simulink library "inp"  (Simulink 8.2)
settings: Solver=ode45  SolverMode=SingleTasking  StartTime=0.0  StopTime=10.0  RelTol=1e-3
undefined symbols (not defined in the file or in its folder): C_TICK_TIME_S, ENUM_FAULTSTATE_FAIL, ENUM_FAULTSTATE_INDETERMINATE, ENUM_FAULTSTATE_PASS, ENUM_KEYSWITCH_ON, FALSE, KINP_ABS1CANCommuOk_flg_ovrdflg, KINP_ABS1CANCommuOk_flg_ovrdval, KINP_ABS1CANPeriodTime_s, KINP_ABS1CANRolCntOk_flg_ovrdflg, KINP_ABS1CANRolCntOk_flg_ovrdval, KINP_ABS2CANChkSumOk_flg_ovrdflg, KINP_ABS2CANChkSumOk_flg_ovrdval, KINP_ABS2CANCommuOk_flg_ovrdflg, KINP_ABS2CANCommuOk_flg_ovrdval, KINP_ABS2CANPeriodTime_s, KINP_ABS2CANRolCntOk_flg_ovrdflg, KINP_ABS2CANRolCntOk_flg_ovrdval, KINP_ABS3CANCommuOk_flg_ovrdflg, KINP_ABS3CANCommuOk_flg_ovrdval, KINP_ABS4CANCommuOk_flg_ovrdflg, KINP_ABS4CANCommuOk_flg_ovrdval, KINP_ABSActive_flg_defval, KINP_ABSActive_flg_invalid, KINP_ABSActive_flg_ovrdflg, KINP_ABSActive_flg_ovrdval, KINP_ABSActive_flg_setvalid, KINP_ABSActive_flg_srvcflg, KINP_ABSActive_flg_srvcval, KINP_ABSCANAlive_flg_ovrdflg, KINP_ABSCANAlive_flg_ovrdval, KINP_ABSCANCommuOk_flg_ovrdflg, KINP_ABSCANCommuOk_flg_ovrdval, KINP_ABSCANValidOk_flg_ovrdflg, KINP_ABSCANValidOk_flg_ovrdval, KINP_ABSFault_flg_defval, KINP_ABSFault_flg_invalid, KINP_ABSFault_flg_ovrdflg, KINP_ABSFault_flg_ovrdval, KINP_ABSFault_flg_setvalid, KINP_ABSFault_flg_srvcflg, KINP_ABSFault_flg_srvcval, KINP_AC1CANCommuOk_flg_ovrdflg, KINP_AC1CANCommuOk_flg_ovrdval, KINP_ACCANAlive_flg_ovrdflg, KINP_ACCANAlive_flg_ovrdval, KINP_ACCANCommuOk_flg_ovrdflg, KINP_ACCANCommuOk_flg_ovrdval, KINP_ACCANValidOk_flg_ovrdflg, KINP_ACCANValidOk_flg_ovrdval, KINP_ACCCancelH_flg_defval, KINP_ACCCancelH_flg_invalid, KINP_ACCCancelH_flg_ovrdflg, KINP_ACCCancelH_flg_ovrdval, KINP_ACCCancelH_flg_setvalid, KINP_ACCCancelH_flg_srvcflg, KINP_ACCCancelH_flg_srvcval, KINP_AccelPedalPosn_pct_defval, KINP_AccelPedalPosn_pct_gain, KINP_AccelPedalPosn_pct_invalid ... (+1866)
libraries: inp x192, hcu_lib x34, simulink x33
(part 12 of 21)

== system inp/INP/EPM_signals/TimeToLaneCrossingRight ==
  signal_in : Inport
  msg_valid : Inport  Port=2
  Active_fault_flg : Inport  Port=3
  can_signal_process_wio_validity : inp/can_signal_process_wio_validity
  conv_gain_offset : Subsystem
  defval : Constant  Value=KINP_TLCR_s_defval
  invalid : Constant  Value=KINP_TLCR_s_invalid
  min_max_limit : Subsystem
  ovrdflg : Constant  Value=KINP_TLCR_s_ovrdflg
  ovrdval : Constant  Value=KINP_TLCR_s_ovrdval
  setvalid : Constant  Value=KINP_TLCR_s_setvalid
  srvcflg : Constant  Value=KINP_TLCR_s_srvcflg
  srvcval : Constant  Value=KINP_TLCR_s_srvcval
  signal_out : Outport
  signal_valid_out : Outport  Port=2
  wires:
  Active_fault_flg/1 -> can_signal_process_wio_validity/3
  srvcval/1 -> can_signal_process_wio_validity/10
  srvcflg/1 -> can_signal_process_wio_validity/9
  ovrdval/1 -> can_signal_process_wio_validity/8
  ovrdflg/1 -> can_signal_process_wio_validity/7
  setvalid/1 -> can_signal_process_wio_validity/6
  min_max_limit/1(out) -> signal_out/1
  conv_gain_offset/1(out) -> can_signal_process_wio_validity/1
  invalid/1 -> can_signal_process_wio_validity/5
  defval/1 -> can_signal_process_wio_validity/4
  msg_valid/1 -> can_signal_process_wio_validity/2
  signal_in/1 -> conv_gain_offset/1(in)
  can_signal_process_wio_validity/2 -> signal_valid_out/1
  can_signal_process_wio_validity/1 -> min_max_limit/1(in)

== system inp/INP/EPM_signals/ViewRangeStartL ==
  signal_in : Inport
  msg_valid : Inport  Port=2
  Active_fault_flg : Inport  Port=3
  can_signal_process_wio_validity : inp/can_signal_process_wio_validity
  conv_gain_offset : Subsystem
  defval : Constant  Value=KINP_ViewRangeStartL_m_defval
  invalid : Constant  Value=KINP_ViewRangeStartL_m_invalid
  min_max_limit : Subsystem
  ovrdflg : Constant  Value=KINP_ViewRangeStartL_m_ovrdflg
  ovrdval : Constant  Value=KINP_ViewRangeStartL_m_ovrdval
  setvalid : Constant  Value=KINP_ViewRangeStartL_m_setvalid
  srvcflg : Constant  Value=KINP_ViewRangeStartL_m_srvcflg
  srvcval : Constant  Value=KINP_ViewRangeStartL_m_srvcval
  signal_out : Outport
  signal_valid_out : Outport  Port=2
  wires:
  Active_fault_flg/1 -> can_signal_process_wio_validity/3
  srvcval/1 -> can_signal_process_wio_validity/10
  srvcflg/1 -> can_signal_process_wio_validity/9
  ovrdval/1 -> can_signal_process_wio_validity/8
  ovrdflg/1 -> can_signal_process_wio_validity/7
  setvalid/1 -> can_signal_process_wio_validity/6
  min_max_limit/1(out) -> signal_out/1
  conv_gain_offset/1(out) -> can_signal_process_wio_validity/1
  invalid/1 -> can_signal_process_wio_validity/5
  defval/1 -> can_signal_process_wio_validity/4
  msg_valid/1 -> can_signal_process_wio_validity/2
  signal_in/1 -> conv_gain_offset/1(in)
  can_signal_process_wio_validity/2 -> signal_valid_out/1
  can_signal_process_wio_validity/1 -> min_max_limit/1(in)

== system inp/INP/EPM_signals/ViewRangeStartR ==
  signal_in : Inport
  msg_valid : Inport  Port=2
  Active_fault_flg : Inport  Port=3
  can_signal_process_wio_validity : inp/can_signal_process_wio_validity
  conv_gain_offset : Subsystem
  defval : Constant  Value=KINP_ViewRangeStartR_m_defval
  invalid : Constant  Value=KINP_ViewRangeStartR_m_invalid
  min_max_limit : Subsystem
  ovrdflg : Constant  Value=KINP_ViewRangeStartR_m_ovrdflg
  ovrdval : Constant  Value=KINP_ViewRangeStartR_m_ovrdval
  setvalid : Constant  Value=KINP_ViewRangeStartR_m_setvalid
  srvcflg : Constant  Value=KINP_ViewRangeStartR_m_srvcflg
  srvcval : Constant  Value=KINP_ViewRangeStartR_m_srvcval
  signal_out : Outport
  signal_valid_out : Outport  Port=2
  wires:
  can_signal_process_wio_validity/1 -> min_max_limit/1(in)
  can_signal_process_wio_validity/2 -> signal_valid_out/1
  signal_in/1 -> conv_gain_offset/1(in)
  msg_valid/1 -> can_signal_process_wio_validity/2
  defval/1 -> can_signal_process_wio_validity/4
  invalid/1 -> can_signal_process_wio_validity/5
  conv_gain_offset/1(out) -> can_signal_process_wio_validity/1
  min_max_limit/1(out) -> signal_out/1
  setvalid/1 -> can_signal_process_wio_validity/6
  ovrdflg/1 -> can_signal_process_wio_validity/7
  ovrdval/1 -> can_signal_process_wio_validity/8
  srvcflg/1 -> can_signal_process_wio_validity/9
  srvcval/1 -> can_signal_process_wio_validity/10
  Active_fault_flg/1 -> can_signal_process_wio_validity/3

== system inp/INP/EPS_signals/AckCurStrAngReq ==
  signal_in : Inport
  signal_valid_in : Inport  Port=2
  msg_valid : Inport  Port=3
  Active_fault_flg : Inport  Port=4
  can_signal_process_with_validity : inp/can_signal_process_with_validity
  conv_gain_offset : Subsystem
  defval : Constant  Value=KINP_AckCurStrAngReq_deg_defval
  invalid : Constant  Value=KINP_AckCurStrAngReq_deg_invalid
  min_max_limit : Subsystem
  ovrdflg : Constant  Value=KINP_AckCurStrAngReq_deg_ovrdflg
  ovrdval : Constant  Value=KINP_AckCurStrAngReq_deg_ovrdval
  setvalid : Constant  Value=KINP_AckCurStrAngReq_deg_setvalid
  srvcflg : Constant  Value=KINP_AckCurStrAngReq_deg_srvcflg
  srvcval : Constant  Value=KINP_AckCurStrAngReq_deg_srvcval
  signal_out : Outport
  signal_valid_out : Outport  Port=2
  raw_signal_valid_out : Outport  Port=3
  wires:
  can_signal_process_with_validity/3 -> raw_signal_valid_out/1
  Active_fault_flg/1 -> can_signal_process_with_validity/4
  srvcval/1 -> can_signal_process_with_validity/12
  srvcflg/1 -> can_signal_process_with_validity/11
  ovrdval/1 -> can_signal_process_with_validity/10
  ovrdflg/1 -> can_signal_process_with_validity/9
  setvalid/1 -> can_signal_process_with_validity/8
  min_max_limit/1(out) -> signal_out/1
  conv_gain_offset/1(out) -> can_signal_process_with_validity/1
  invalid/1 -> can_signal_process_with_validity/7
  defval/1 -> can_signal_process_with_validity/6
  msg_valid/1 -> can_signal_process_with_validity/5, can_signal_process_with_validity/3
  signal_valid_in/1 -> can_signal_process_with_validity/2
  signal_in/1 -> conv_gain_offset/1(in)
  can_signal_process_with_validity/2 -> signal_valid_out/1
  can_signal_process_with_validity/1 -> min_max_limit/1(in)

== system inp/INP/EPS_signals/AckCurStrAngReqApplable ==
  signal_in : Inport
  msg_valid : Inport  Port=2
  Active_fault_flg : Inport  Port=3
  can_signal_process_wio_validity : inp/can_signal_process_wio_validity
  defval : Constant  Value=KINP_AckCurStrAngReqApplable_flg_defval
  invalid : Constant  Value=KINP_AckCurStrAngReqApplable_flg_invalid
  ovrdflg : Constant  Value=KINP_AckCurStrAngReqApplable_flg_ovrdflg
  ovrdval : Constant  Value=KINP_AckCurStrAngReqApplable_flg_ovrdval
  setvalid : Constant  Value=KINP_AckCurStrAngReqApplable_flg_setvalid
  srvcflg : Constant  Value=KINP_AckCurStrAngReqApplable_flg_srvcflg
  srvcval : Constant  Value=KINP_AckCurStrAngReqApplable_flg_srvcval
  signal_out : Outport
  signal_valid_out : Outport  Port=2
  wires:
  Active_fault_flg/1 -> can_signal_process_wio_validity/3
  srvcval/1 -> can_signal_process_wio_validity/10
  srvcflg/1 -> can_signal_process_wio_validity/9
  ovrdval/1 -> can_signal_process_wio_validity/8
  ovrdflg/1 -> can_signal_process_wio_validity/7
  setvalid/1 -> can_signal_process_wio_validity/6
  invalid/1 -> can_signal_process_wio_validity/5
  defval/1 -> can_signal_process_wio_validity/4
  msg_valid/1 -> can_signal_process_wio_validity/2
  signal_in/1 -> can_signal_process_wio_validity/1
  can_signal_process_wio_validity/2 -> signal_valid_out/1
  can_signal_process_wio_validity/1 -> signal_out/1

== system inp/INP/EPS_signals/AckCurStrTqReq ==
  signal_in : Inport
  signal_valid_in : Inport  Port=2
  msg_valid : Inport  Port=3
  Active_fault_flg : Inport  Port=4
  can_signal_process_with_validity : inp/can_signal_process_with_validity
  conv_gain_offset : Subsystem
  defval : Constant  Value=KINP_AckCurStrTqReq_Nm_defval
  invalid : Constant  Value=KINP_AckCurStrTqReq_Nm_invalid
  min_max_limit : Subsystem
  ovrdflg : Constant  Value=KINP_AckCurStrTqReq_Nm_ovrdflg
  ovrdval : Constant  Value=KINP_AckCurStrTqReq_Nm_ovrdval
  setvalid : Constant  Value=KINP_AckCurStrTqReq_Nm_setvalid
  srvcflg : Constant  Value=KINP_AckCurStrTqReq_Nm_srvcflg
  srvcval : Constant  Value=KINP_AckCurStrTqReq_Nm_srvcval
  signal_out : Outport
  signal_valid_out : Outport  Port=2
  raw_signal_valid_out : Outport  Port=3
  wires:
  can_signal_process_with_validity/1 -> min_max_limit/1(in)
  can_signal_process_with_validity/2 -> signal_valid_out/1
  signal_in/1 -> conv_gain_offset/1(in)
  signal_valid_in/1 -> can_signal_process_with_validity/2
  msg_valid/1 -> can_signal_process_with_validity/3, can_signal_process_with_validity/5
  defval/1 -> can_signal_process_with_validity/6
  invalid/1 -> can_signal_process_with_validity/7
  conv_gain_offset/1(out) -> can_signal_process_with_validity/1
  min_max_limit/1(out) -> signal_out/1
  setvalid/1 -> can_signal_process_with_validity/8
  ovrdflg/1 -> can_signal_process_with_validity/9
  ovrdval/1 -> can_signal_process_with_validity/10
  srvcflg/1 -> can_signal_process_with_validity/11
  srvcval/1 -> can_signal_process_with_validity/12
  Active_fault_flg/1 -> can_signal_process_with_validity/4
  can_signal_process_with_validity/3 -> raw_signal_valid_out/1

== system inp/INP/EPS_signals/AckCurStrTqReqApplable ==
  signal_in : Inport
  msg_valid : Inport  Port=2
  Active_fault_flg : Inport  Port=3
  can_signal_process_wio_validity : inp/can_signal_process_wio_validity
  defval : Constant  Value=KINP_AckCurStrTqReqApplable_flg_defval
  invalid : Constant  Value=KINP_AckCurStrTqReqApplable_flg_invalid
  ovrdflg : Constant  Value=KINP_AckCurStrTqReqApplable_flg_ovrdflg
  ovrdval : Constant  Value=KINP_AckCurStrTqReqApplable_flg_ovrdval
  setvalid : Constant  Value=KINP_AckCurStrTqReqApplable_flg_setvalid
  srvcflg : Constant  Value=KINP_AckCurStrTqReqApplable_flg_srvcflg
  srvcval : Constant  Value=KINP_AckCurStrTqReqApplable_flg_srvcval
  signal_out : Outport
  signal_valid_out : Outport  Port=2
  wires:
  can_signal_process_wio_validity/1 -> signal_out/1
  can_signal_process_wio_validity/2 -> signal_valid_out/1
  signal_in/1 -> can_signal_process_wio_validity/1
  msg_valid/1 -> can_signal_process_wio_validity/2
  defval/1 -> can_signal_process_wio_validity/4
  invalid/1 -> can_signal_process_wio_validity/5
  setvalid/1 -> can_signal_process_wio_validity/6
  ovrdflg/1 -> can_signal_process_wio_validity/7
  ovrdval/1 -> can_signal_process_wio_validity/8
  srvcflg/1 -> can_signal_process_wio_validity/9
  srvcval/1 -> can_signal_process_wio_validity/10
  Active_fault_flg/1 -> can_signal_process_wio_validity/3

== system inp/INP/EPS_signals/EPASMILReq ==
  signal_in : Inport
  msg_valid : Inport  Port=2
  Active_fault_flg : Inport  Port=3
  can_signal_process_wio_validity : inp/can_signal_process_wio_validity
  defval : Constant  Value=KINP_EPASMILReq_flg_defval
  invalid : Constant  Value=KINP_EPASMILReq_flg_invalid
  ovrdflg : Constant  Value=KINP_EPASMILReq_flg_ovrdflg
  ovrdval : Constant  Value=KINP_EPASMILReq_flg_ovrdval
  setvalid : Constant  Value=KINP_EPASMILReq_flg_setvalid
  srvcflg : Constant  Value=KINP_EPASMILReq_flg_srvcflg
  srvcval : Constant  Value=KINP_EPASMILReq_flg_srvcval
  signal_out : Outport
  signal_valid_out : Outport  Port=2
  wires:
  Active_fault_flg/1 -> can_signal_process_wio_validity/3
  srvcval/1 -> can_signal_process_wio_validity/10
  srvcflg/1 -> can_signal_process_wio_validity/9
  ovrdval/1 -> can_signal_process_wio_validity/8
  ovrdflg/1 -> can_signal_process_wio_validity/7
  setvalid/1 -> can_signal_process_wio_validity/6
  invalid/1 -> can_signal_process_wio_validity/5
  defval/1 -> can_signal_process_wio_validity/4
  msg_valid/1 -> can_signal_process_wio_validity/2
  signal_in/1 -> can_signal_process_wio_validity/1
  can_signal_process_wio_validity/2 -> signal_valid_out/1
  can_signal_process_wio_validity/1 -> signal_out/1

== system inp/INP/EPS_signals/EPSBattVolt ==
  signal_in : Inport
  signal_valid_in : Inport  Port=2
  msg_valid : Inport  Port=3
  Active_fault_flg : Inport  Port=4
  can_signal_process_with_validity : inp/can_signal_process_with_validity
  conv_gain_offset : Subsystem
  defval : Constant  Value=KINP_EPSBattVolt_V_defval
  invalid : Constant  Value=KINP_EPSBattVolt_V_invalid
  min_max_limit : Subsystem
  ovrdflg : Constant  Value=KINP_EPSBattVolt_V_ovrdflg
  ovrdval : Constant  Value=KINP_EPSBattVolt_V_ovrdval
  setvalid : Constant  Value=KINP_EPSBattVolt_V_setvalid
  srvcflg : Constant  Value=KINP_EPSBattVolt_V_srvcflg
  srvcval : Constant  Value=KINP_EPSBattVolt_V_srvcval
  signal_out : Outport
  signal_valid_out : Outport  Port=2
  raw_signal_valid_out : Outport  Port=3
  wires:
  can_signal_process_with_validity/3 -> raw_signal_valid_out/1
  Active_fault_flg/1 -> can_signal_process_with_validity/4
  srvcval/1 -> can_signal_process_with_validity/12
  srvcflg/1 -> can_signal_process_with_validity/11
  ovrdval/1 -> can_signal_process_with_validity/10
  ovrdflg/1 -> can_signal_process_with_validity/9
  setvalid/1 -> can_signal_process_with_validity/8
  min_max_limit/1(out) -> signal_out/1
  conv_gain_offset/1(out) -> can_signal_process_with_validity/1
  invalid/1 -> can_signal_process_with_validity/7
  defval/1 -> can_signal_process_with_validity/6
  msg_valid/1 -> can_signal_process_with_validity/5, can_signal_process_with_validity/3
  signal_valid_in/1 -> can_signal_process_with_validity/2
  signal_in/1 -> conv_gain_offset/1(in)
  can_signal_process_with_validity/2 -> signal_valid_out/1
  can_signal_process_with_validity/1 -> min_max_limit/1(in)

== system inp/INP/EPS_signals/EPSElectPWR ==
  signal_in : Inport
  msg_valid : Inport  Port=2
  Active_fault_flg : Inport  Port=3
  can_signal_process_wio_validity : inp/can_signal_process_wio_validity
  conv_gain_offset : Subsystem
  defval : Constant  Value=KINP_EPSElectPWR_A_defval
  invalid : Constant  Value=KINP_EPSElectPWR_A_invalid
  min_max_limit : Subsystem
  ovrdflg : Constant  Value=KINP_EPSElectPWR_A_ovrdflg
  ovrdval : Constant  Value=KINP_EPSElectPWR_A_ovrdval
  setvalid : Constant  Value=KINP_EPSElectPWR_A_setvalid
  srvcflg : Constant  Value=KINP_EPSElectPWR_A_srvcflg
  srvcval : Constant  Value=KINP_EPSElectPWR_A_srvcval
  signal_out : Outport
  signal_valid_out : Outport  Port=2
  wires:
  can_signal_process_wio_validity/1 -> min_max_limit/1(in)
  can_signal_process_wio_validity/2 -> signal_valid_out/1
  signal_in/1 -> conv_gain_offset/1(in)
  msg_valid/1 -> can_signal_process_wio_validity/2
  defval/1 -> can_signal_process_wio_validity/4
  invalid/1 -> can_signal_process_wio_validity/5
  conv_gain_offset/1(out) -> can_signal_process_wio_validity/1
  min_max_limit/1(out) -> signal_out/1
  setvalid/1 -> can_signal_process_wio_validity/6
  ovrdflg/1 -> can_signal_process_wio_validity/7
  ovrdval/1 -> can_signal_process_wio_validity/8
  srvcflg/1 -> can_signal_process_wio_validity/9
  srvcval/1 -> can_signal_process_wio_validity/10
  Active_fault_flg/1 -> can_signal_process_wio_validity/3

== system inp/INP/EPS_signals/EPSFailed ==
  signal_in : Inport
  msg_valid : Inport  Port=2
  Active_fault_flg : Inport  Port=3
  can_signal_process_wio_validity : inp/can_signal_process_wio_validity
  defval : Constant  Value=KINP_EPSFailed_flg_defval
  invalid : Constant  Value=KINP_EPSFailed_flg_invalid
  ovrdflg : Constant  Value=KINP_EPSFailed_flg_ovrdflg
  ovrdval : Constant  Value=KINP_EPSFailed_flg_ovrdval
  setvalid : Constant  Value=KINP_EPSFailed_flg_setvalid
  srvcflg : Constant  Value=KINP_EPSFailed_flg_srvcflg
  srvcval : Constant  Value=KINP_EPSFailed_flg_srvcval
  signal_out : Outport
  signal_valid_out : Outport  Port=2
  wires:
  Active_fault_flg/1 -> can_signal_process_wio_validity/3
  srvcval/1 -> can_signal_process_wio_validity/10
  srvcflg/1 -> can_signal_process_wio_validity/9
  ovrdval/1 -> can_signal_process_wio_validity/8
  ovrdflg/1 -> can_signal_process_wio_validity/7
  setvalid/1 -> can_signal_process_wio_validity/6
  invalid/1 -> can_signal_process_wio_validity/5
  defval/1 -> can_signal_process_wio_validity/4
  msg_valid/1 -> can_signal_process_wio_validity/2
  signal_in/1 -> can_signal_process_wio_validity/1
  can_signal_process_wio_validity/2 -> signal_valid_out/1
  can_signal_process_wio_validity/1 -> signal_out/1

== system inp/INP/EPS_signals/RelSteerAngle ==
  signal_in : Inport
  signal_valid_in : Inport  Port=2
  msg_valid : Inport  Port=3
  Active_fault_flg : Inport  Port=4
  can_signal_process_with_validity : inp/can_signal_process_with_validity
  conv_gain_offset : Subsystem
  defval : Constant  Value=KINP_RelSteerAngle_deg_defval
  invalid : Constant  Value=KINP_RelSteerAngle_deg_invalid
  min_max_limit : Subsystem
  ovrdflg : Constant  Value=KINP_RelSteerAngle_deg_ovrdflg
  ovrdval : Constant  Value=KINP_RelSteerAngle_deg_ovrdval
  setvalid : Constant  Value=KINP_RelSteerAngle_deg_setvalid
  srvcflg : Constant  Value=KINP_RelSteerAngle_deg_srvcflg
  srvcval : Constant  Value=KINP_RelSteerAngle_deg_srvcval
  signal_out : Outport
  signal_valid_out : Outport  Port=2
  raw_signal_valid_out : Outport  Port=3
  wires:
  can_signal_process_with_validity/1 -> min_max_limit/1(in)
  can_signal_process_with_validity/2 -> signal_valid_out/1
  signal_in/1 -> conv_gain_offset/1(in)
  signal_valid_in/1 -> can_signal_process_with_validity/2
  msg_valid/1 -> can_signal_process_with_validity/3, can_signal_process_with_validity/5
  defval/1 -> can_signal_process_with_validity/6
  invalid/1 -> can_signal_process_with_validity/7
  conv_gain_offset/1(out) -> can_signal_process_with_validity/1
  min_max_limit/1(out) -> signal_out/1
  setvalid/1 -> can_signal_process_with_validity/8
  ovrdflg/1 -> can_signal_process_with_validity/9
  ovrdval/1 -> can_signal_process_with_validity/10
  srvcflg/1 -> can_signal_process_with_validity/11
  srvcval/1 -> can_signal_process_with_validity/12
  Active_fault_flg/1 -> can_signal_process_with_validity/4
  can_signal_process_with_validity/3 -> raw_signal_valid_out/1

== system inp/INP/EPS_signals/SteerTorque ==
  signal_in : Inport
  signal_valid_in : Inport  Port=2
  msg_valid : Inport  Port=3
  Active_fault_flg : Inport  Port=4
  can_signal_process_with_validity : inp/can_signal_process_with_validity
  conv_gain_offset : Subsystem
  defval : Constant  Value=KINP_SteerTorque_Nm_defval
  invalid : Constant  Value=KINP_SteerTorque_Nm_invalid
  min_max_limit : Subsystem
  ovrdflg : Constant  Value=KINP_SteerTorque_Nm_ovrdflg
  ovrdval : Constant  Value=KINP_SteerTorque_Nm_ovrdval
  setvalid : Constant  Value=KINP_SteerTorque_Nm_setvalid
  srvcflg : Constant  Value=KINP_SteerTorque_Nm_srvcflg
  srvcval : Constant  Value=KINP_SteerTorque_Nm_srvcval
  signal_out : Outport
  signal_valid_out : Outport  Port=2
  raw_signal_valid_out : Outport  Port=3
  wires:
  can_signal_process_with_validity/3 -> raw_signal_valid_out/1
  Active_fault_flg/1 -> can_signal_process_with_validity/4
  srvcval/1 -> can_signal_process_with_validity/12
  srvcflg/1 -> can_signal_process_with_validity/11
  ovrdval/1 -> can_signal_process_with_validity/10
  ovrdflg/1 -> can_signal_process_with_validity/9
  setvalid/1 -> can_signal_process_with_validity/8
  min_max_limit/1(out) -> signal_out/1
  conv_gain_offset/1(out) -> can_signal_process_with_validity/1
  invalid/1 -> can_signal_process_with_validity/7
  defval/1 -> can_signal_process_with_validity/6
  msg_valid/1 -> can_signal_process_with_validity/5, can_signal_process_with_validity/3
  signal_valid_in/1 -> can_signal_process_with_validity/2
  signal_in/1 -> conv_gain_offset/1(in)
  can_signal_process_with_validity/2 -> signal_valid_out/1
  can_signal_process_with_validity/1 -> min_max_limit/1(in)

== system inp/INP/EPS_signals/SteeringAngle ==
  signal_in : Inport
  signal_valid_in : Inport  Port=2
  msg_valid : Inport  Port=3
  Active_fault_flg : Inport  Port=4
  can_signal_process_with_validity : inp/can_signal_process_with_validity
  conv_gain_offset : Subsystem
  defval : Constant  Value=KINP_SteerAngle_deg_defval
  invalid : Constant  Value=KINP_SteerAngle_deg_invalid
  min_max_limit : Subsystem
  ovrdflg : Constant  Value=KINP_SteerAngle_deg_ovrdflg
  ovrdval : Constant  Value=KINP_SteerAngle_deg_ovrdval
  setvalid : Constant  Value=KINP_SteerAngle_deg_setvalid
  srvcflg : Constant  Value=KINP_SteerAngle_deg_srvcflg
  srvcval : Constant  Value=KINP_SteerAngle_deg_srvcval
  signal_out : Outport
  signal_valid_out : Outport  Port=2
  raw_signal_valid_out : Outport  Port=3
  wires:
  can_signal_process_with_validity/1 -> min_max_limit/1(in)
  can_signal_process_with_validity/2 -> signal_valid_out/1
  signal_in/1 -> conv_gain_offset/1(in)
  signal_valid_in/1 -> can_signal_process_with_validity/2
  msg_valid/1 -> can_signal_process_with_validity/3, can_signal_process_with_validity/5
  defval/1 -> can_signal_process_with_validity/6
  invalid/1 -> can_signal_process_with_validity/7
  conv_gain_offset/1(out) -> can_signal_process_with_validity/1
  min_max_limit/1(out) -> signal_out/1
  setvalid/1 -> can_signal_process_with_validity/8
  ovrdflg/1 -> can_signal_process_with_validity/9
  ovrdval/1 -> can_signal_process_with_validity/10
  srvcflg/1 -> can_signal_process_with_validity/11
  srvcval/1 -> can_signal_process_with_validity/12
  Active_fault_flg/1 -> can_signal_process_with_validity/4
  can_signal_process_with_validity/3 -> raw_signal_valid_out/1

== system inp/INP/EPS_signals/SteeringSpeed ==
  signal_in : Inport
  signal_valid_in : Inport  Port=2
  msg_valid : Inport  Port=3
  Active_fault_flg : Inport  Port=4
  can_signal_process_with_validity : inp/can_signal_process_with_validity
  conv_gain_offset : Subsystem
  defval : Constant  Value=KINP_SteerSpeed_degps_defval
  invalid : Constant  Value=KINP_SteerSpeed_degps_invalid
  min_max_limit : Subsystem
  ovrdflg : Constant  Value=KINP_SteerSpeed_degps_ovrdflg
  ovrdval : Constant  Value=KINP_SteerSpeed_degps_ovrdval
  setvalid : Constant  Value=KINP_SteerSpeed_degps_setvalid
  srvcflg : Constant  Value=KINP_SteerSpeed_degps_srvcflg
  srvcval : Constant  Value=KINP_SteerSpeed_degps_srvcval
  signal_out : Outport
  signal_valid_out : Outport  Port=2
  raw_signal_valid_out : Outport  Port=3
  wires:
  can_signal_process_with_validity/3 -> raw_signal_valid_out/1
  Active_fault_flg/1 -> can_signal_process_with_validity/4
  srvcval/1 -> can_signal_process_with_validity/12
  srvcflg/1 -> can_signal_process_with_validity/11
  ovrdval/1 -> can_signal_process_with_validity/10
  ovrdflg/1 -> can_signal_process_with_validity/9
  setvalid/1 -> can_signal_process_with_validity/8
  min_max_limit/1(out) -> signal_out/1
  conv_gain_offset/1(out) -> can_signal_process_with_validity/1
  invalid/1 -> can_signal_process_with_validity/7
  defval/1 -> can_signal_process_with_validity/6
  msg_valid/1 -> can_signal_process_with_validity/5, can_signal_process_with_validity/3
  signal_valid_in/1 -> can_signal_process_with_validity/2
  signal_in/1 -> conv_gain_offset/1(in)
  can_signal_process_with_validity/2 -> signal_valid_out/1
  can_signal_process_with_validity/1 -> min_max_limit/1(in)

== system inp/INP/HMI_signals/ACCCancelH ==
  signal_in : Inport
  msg_valid : Inport  Port=2
  Active_fault_flg : Inport  Port=3
  can_signal_process_wio_validity : inp/can_signal_process_wio_validity
  defval : Constant  Value=KINP_ACCCancelH_flg_defval
  invalid : Constant  Value=KINP_ACCCancelH_flg_invalid
  ovrdflg : Constant  Value=KINP_ACCCancelH_flg_ovrdflg
  ovrdval : Constant  Value=KINP_ACCCancelH_flg_ovrdval
  setvalid : Constant  Value=KINP_ACCCancelH_flg_setvalid
  srvcflg : Constant  Value=KINP_ACCCancelH_flg_srvcflg
  srvcval : Constant  Value=KINP_ACCCancelH_flg_srvcval
  signal_out : Outport
  signal_valid_out : Outport  Port=2
  wires:
  Active_fault_flg/1 -> can_signal_process_wio_validity/3
  srvcval/1 -> can_signal_process_wio_validity/10
  srvcflg/1 -> can_signal_process_wio_validity/9
  ovrdval/1 -> can_signal_process_wio_validity/8
  ovrdflg/1 -> can_signal_process_wio_validity/7
  setvalid/1 -> can_signal_process_wio_validity/6
  invalid/1 -> can_signal_process_wio_validity/5
  defval/1 -> can_signal_process_wio_validity/4
  msg_valid/1 -> can_signal_process_wio_validity/2
  signal_in/1 -> can_signal_process_wio_validity/1
  can_signal_process_wio_validity/2 -> signal_valid_out/1
  can_signal_process_wio_validity/1 -> signal_out/1

== system inp/INP/HMI_signals/ACCOnH ==
  signal_in : Inport
  msg_valid : Inport  Port=2
  Active_fault_flg : Inport  Port=3
  can_signal_process_wio_validity : inp/can_signal_process_wio_validity
  defval : Constant  Value=KINP_ACCOnH_flg_defval
  invalid : Constant  Value=KINP_ACCOnH_flg_invalid
  ovrdflg : Constant  Value=KINP_ACCOnH_flg_ovrdflg
  ovrdval : Constant  Value=KINP_ACCOnH_flg_ovrdval
  setvalid : Constant  Value=KINP_ACCOnH_flg_setvalid
  srvcflg : Constant  Value=KINP_ACCOnH_flg_srvcflg
  srvcval : Constant  Value=KINP_ACCOnH_flg_srvcval
  signal_out : Outport
  signal_valid_out : Outport  Port=2
  wires:
  can_signal_process_wio_validity/1 -> signal_out/1
  can_signal_process_wio_validity/2 -> signal_valid_out/1
  signal_in/1 -> can_signal_process_wio_validity/1
  msg_valid/1 -> can_signal_process_wio_validity/2
  defval/1 -> can_signal_process_wio_validity/4
  invalid/1 -> can_signal_process_wio_validity/5
  setvalid/1 -> can_signal_process_wio_validity/6
  ovrdflg/1 -> can_signal_process_wio_validity/7
  ovrdval/1 -> can_signal_process_wio_validity/8
  srvcflg/1 -> can_signal_process_wio_validity/9
  srvcval/1 -> can_signal_process_wio_validity/10
  Active_fault_flg/1 -> can_signal_process_wio_validity/3

== system inp/INP/HMI_signals/ACCResSpdIncH ==
  signal_in : Inport
  msg_valid : Inport  Port=2
  Active_fault_flg : Inport  Port=3
  can_signal_process_wio_validity : inp/can_signal_process_wio_validity
  defval : Constant  Value=KINP_ACCResSpdIncH_flg_defval
  invalid : Constant  Value=KINP_ACCResSpdIncH_flg_invalid
  ovrdflg : Constant  Value=KINP_ACCResSpdIncH_flg_ovrdflg
  ovrdval : Constant  Value=KINP_ACCResSpdIncH_flg_ovrdval
  setvalid : Constant  Value=KINP_ACCResSpdIncH_flg_setvalid
  srvcflg : Constant  Value=KINP_ACCResSpdIncH_flg_srvcflg
  srvcval : Constant  Value=KINP_ACCResSpdIncH_flg_srvcval
  signal_out : Outport
  signal_valid_out : Outport  Port=2
  wires:
  can_signal_process_wio_validity/1 -> signal_out/1
  can_signal_process_wio_validity/2 -> signal_valid_out/1
  signal_in/1 -> can_signal_process_wio_validity/1
  msg_valid/1 -> can_signal_process_wio_validity/2
  defval/1 -> can_signal_process_wio_validity/4
  invalid/1 -> can_signal_process_wio_validity/5
  setvalid/1 -> can_signal_process_wio_validity/6
  ovrdflg/1 -> can_signal_process_wio_validity/7
  ovrdval/1 -> can_signal_process_wio_validity/8
  srvcflg/1 -> can_signal_process_wio_validity/9
  srvcval/1 -> can_signal_process_wio_validity/10
  Active_fault_flg/1 -> can_signal_process_wio_validity/3

== system inp/INP/HMI_signals/ACCSetSpdDecH ==
  signal_in : Inport
  msg_valid : Inport  Port=2
  Active_fault_flg : Inport  Port=3
  can_signal_process_wio_validity : inp/can_signal_process_wio_validity
  defval : Constant  Value=KINP_ACCSetSpdDecH_flg_defval
  invalid : Constant  Value=KINP_ACCSetSpdDecH_flg_invalid
  ovrdflg : Constant  Value=KINP_ACCSetSpdDecH_flg_ovrdflg
  ovrdval : Constant  Value=KINP_ACCSetSpdDecH_flg_ovrdval
  setvalid : Constant  Value=KINP_ACCSetSpdDecH_flg_setvalid
  srvcflg : Constant  Value=KINP_ACCSetSpdDecH_flg_srvcflg
  srvcval : Constant  Value=KINP_ACCSetSpdDecH_flg_srvcval
  signal_out : Outport
  signal_valid_out : Outport  Port=2
  wires:
  Active_fault_flg/1 -> can_signal_process_wio_validity/3
  srvcval/1 -> can_signal_process_wio_validity/10
  srvcflg/1 -> can_signal_process_wio_validity/9
  ovrdval/1 -> can_signal_process_wio_validity/8
  ovrdflg/1 -> can_signal_process_wio_validity/7
  setvalid/1 -> can_signal_process_wio_validity/6
  invalid/1 -> can_signal_process_wio_validity/5
  defval/1 -> can_signal_process_wio_validity/4
  msg_valid/1 -> can_signal_process_wio_validity/2
  signal_in/1 -> can_signal_process_wio_validity/1
  can_signal_process_wio_validity/2 -> signal_valid_out/1
  can_signal_process_wio_validity/1 -> signal_out/1

== system inp/INP/HMI_signals/ACCSpdDecH ==
  signal_in : Inport
  msg_valid : Inport  Port=2
  Active_fault_flg : Inport  Port=3
  can_signal_process_wio_validity : inp/can_signal_process_wio_validity
  defval : Constant  Value=KINP_ACCSpdDecH_flg_defval
  invalid : Constant  Value=KINP_ACCSpdDecH_flg_invalid
  ovrdflg : Constant  Value=KINP_ACCSpdDecH_flg_ovrdflg
  ovrdval : Constant  Value=KINP_ACCSpdDecH_flg_ovrdval
  setvalid : Constant  Value=KINP_ACCSpdDecH_flg_setvalid
  srvcflg : Constant  Value=KINP_ACCSpdDecH_flg_srvcflg
  srvcval : Constant  Value=KINP_ACCSpdDecH_flg_srvcval
  signal_out : Outport
  signal_valid_out : Outport  Port=2
  wires:
  Active_fault_flg/1 -> can_signal_process_wio_validity/3
  srvcval/1 -> can_signal_process_wio_validity/10
  srvcflg/1 -> can_signal_process_wio_validity/9
  ovrdval/1 -> can_signal_process_wio_validity/8
  ovrdflg/1 -> can_signal_process_wio_validity/7
  setvalid/1 -> can_signal_process_wio_validity/6
  invalid/1 -> can_signal_process_wio_validity/5
  defval/1 -> can_signal_process_wio_validity/4
  msg_valid/1 -> can_signal_process_wio_validity/2
  signal_in/1 -> can_signal_process_wio_validity/1
  can_signal_process_wio_validity/2 -> signal_valid_out/1
  can_signal_process_wio_validity/1 -> signal_out/1

== system inp/INP/HMI_signals/ACCSpdIncH ==
  signal_in : Inport
  msg_valid : Inport  Port=2
  Active_fault_flg : Inport  Port=3
  can_signal_process_wio_validity : inp/can_signal_process_wio_validity
  defval : Constant  Value=KINP_ACCSpdIncH_flg_defval
  invalid : Constant  Value=KINP_ACCSpdIncH_flg_invalid
  ovrdflg : Constant  Value=KINP_ACCSpdIncH_flg_ovrdflg
  ovrdval : Constant  Value=KINP_ACCSpdIncH_flg_ovrdval
  setvalid : Constant  Value=KINP_ACCSpdIncH_flg_setvalid
  srvcflg : Constant  Value=KINP_ACCSpdIncH_flg_srvcflg
  srvcval : Constant  Value=KINP_ACCSpdIncH_flg_srvcval
  signal_out : Outport
  signal_valid_out : Outport  Port=2
  wires:
  can_signal_process_wio_validity/1 -> signal_out/1
  can_signal_process_wio_validity/2 -> signal_valid_out/1
  signal_in/1 -> can_signal_process_wio_validity/1
  msg_valid/1 -> can_signal_process_wio_validity/2
  defval/1 -> can_signal_process_wio_validity/4
  invalid/1 -> can_signal_process_wio_validity/5
  setvalid/1 -> can_signal_process_wio_validity/6
  ovrdflg/1 -> can_signal_process_wio_validity/7
  ovrdval/1 -> can_signal_process_wio_validity/8
  srvcflg/1 -> can_signal_process_wio_validity/9
  srvcval/1 -> can_signal_process_wio_validity/10
  Active_fault_flg/1 -> can_signal_process_wio_validity/3

== system inp/INP/HMI_signals/ACCTimeGapDecH ==
  signal_in : Inport
  msg_valid : Inport  Port=2
  Active_fault_flg : Inport  Port=3
  can_signal_process_wio_validity : inp/can_signal_process_wio_validity
  defval : Constant  Value=KINP_ACCTimeGapDecH_flg_defval
  invalid : Constant  Value=KINP_ACCTimeGapDecH_flg_invalid
  ovrdflg : Constant  Value=KINP_ACCTimeGapDecH_flg_ovrdflg
  ovrdval : Constant  Value=KINP_ACCTimeGapDecH_flg_ovrdval
  setvalid : Constant  Value=KINP_ACCTimeGapDecH_flg_setvalid
  srvcflg : Constant  Value=KINP_ACCTimeGapDecH_flg_srvcflg
  srvcval : Constant  Value=KINP_ACCTimeGapDecH_flg_srvcval
  signal_out : Outport
  signal_valid_out : Outport  Port=2
  wires:
  Active_fault_flg/1 -> can_signal_process_wio_validity/3
  srvcval/1 -> can_signal_process_wio_validity/10
  srvcflg/1 -> can_signal_process_wio_validity/9
  ovrdval/1 -> can_signal_process_wio_validity/8
  ovrdflg/1 -> can_signal_process_wio_validity/7
  setvalid/1 -> can_signal_process_wio_validity/6
  invalid/1 -> can_signal_process_wio_validity/5
  defval/1 -> can_signal_process_wio_validity/4
  msg_valid/1 -> can_signal_process_wio_validity/2
  signal_in/1 -> can_signal_process_wio_validity/1
  can_signal_process_wio_validity/2 -> signal_valid_out/1
  can_signal_process_wio_validity/1 -> signal_out/1

== system inp/INP/HMI_signals/ACCTimeGapIncH ==
  signal_in : Inport
  msg_valid : Inport  Port=2
  Active_fault_flg : Inport  Port=3
  can_signal_process_wio_validity : inp/can_signal_process_wio_validity
  defval : Constant  Value=KINP_ACCTimeGapIncH_flg_defval
  invalid : Constant  Value=KINP_ACCTimeGapIncH_flg_invalid
  ovrdflg : Constant  Value=KINP_ACCTimeGapIncH_flg_ovrdflg
  ovrdval : Constant  Value=KINP_ACCTimeGapIncH_flg_ovrdval
  setvalid : Constant  Value=KINP_ACCTimeGapIncH_flg_setvalid
  srvcflg : Constant  Value=KINP_ACCTimeGapIncH_flg_srvcflg
  srvcval : Constant  Value=KINP_ACCTimeGapIncH_flg_srvcval
  signal_out : Outport
  signal_valid_out : Outport  Port=2
  wires:
  can_signal_process_wio_validity/1 -> signal_out/1
  can_signal_process_wio_validity/2 -> signal_valid_out/1
  signal_in/1 -> can_signal_process_wio_validity/1
  msg_valid/1 -> can_signal_process_wio_validity/2
  defval/1 -> can_signal_process_wio_validity/4
  invalid/1 -> can_signal_process_wio_validity/5
  setvalid/1 -> can_signal_process_wio_validity/6
  ovrdflg/1 -> can_signal_process_wio_validity/7
  ovrdval/1 -> can_signal_process_wio_validity/8
  srvcflg/1 -> can_signal_process_wio_validity/9
  srvcval/1 -> can_signal_process_wio_validity/10
  Active_fault_flg/1 -> can_signal_process_wio_validity/3

== system inp/INP/HMI_signals/AEBEnableH ==
  signal_in : Inport
  msg_valid : Inport  Port=2
  Active_fault_flg : Inport  Port=3
  can_signal_process_wio_validity : inp/can_signal_process_wio_validity
  defval : Constant  Value=KINP_AEBEnableH_flg_defval
  invalid : Constant  Value=KINP_AEBEnableH_flg_invalid
  ovrdflg : Constant  Value=KINP_AEBEnableH_flg_ovrdflg
  ovrdval : Constant  Value=KINP_AEBEnableH_flg_ovrdval
  setvalid : Constant  Value=KINP_AEBEnableH_flg_setvalid
  srvcflg : Constant  Value=KINP_AEBEnableH_flg_srvcflg
  srvcval : Constant  Value=KINP_AEBEnableH_flg_srvcval
  signal_out : Outport
  signal_valid_out : Outport  Port=2
  wires:
  can_signal_process_wio_validity/1 -> signal_out/1
  can_signal_process_wio_validity/2 -> signal_valid_out/1
  signal_in/1 -> can_signal_process_wio_validity/1
  msg_valid/1 -> can_signal_process_wio_validity/2
  defval/1 -> can_signal_process_wio_validity/4
  invalid/1 -> can_signal_process_wio_validity/5
  setvalid/1 -> can_signal_process_wio_validity/6
  ovrdflg/1 -> can_signal_process_wio_validity/7
  ovrdval/1 -> can_signal_process_wio_validity/8
  srvcflg/1 -> can_signal_process_wio_validity/9
  srvcval/1 -> can_signal_process_wio_validity/10
  Active_fault_flg/1 -> can_signal_process_wio_validity/3

== system inp/INP/HMI_signals/CSCEnableH ==
  signal_in : Inport
  msg_valid : Inport  Port=2
  Active_fault_flg : Inport  Port=3
  can_signal_process_wio_validity : inp/can_signal_process_wio_validity
  defval : Constant  Value=KINP_CSCEnableH_flg_defval
  invalid : Constant  Value=KINP_CSCEnableH_flg_invalid
  ovrdflg : Constant  Value=KINP_CSCEnableH_flg_ovrdflg
  ovrdval : Constant  Value=KINP_CSCEnableH_flg_ovrdval
  setvalid : Constant  Value=KINP_CSCEnableH_flg_setvalid
  srvcflg : Constant  Value=KINP_CSCEnableH_flg_srvcflg
  srvcval : Constant  Value=KINP_CSCEnableH_flg_srvcval
  signal_out : Outport
  signal_valid_out : Outport  Port=2
  wires:
  can_signal_process_wio_validity/1 -> signal_out/1
  can_signal_process_wio_validity/2 -> signal_valid_out/1
  signal_in/1 -> can_signal_process_wio_validity/1
  msg_valid/1 -> can_signal_process_wio_validity/2
  defval/1 -> can_signal_process_wio_validity/4
  invalid/1 -> can_signal_process_wio_validity/5
  setvalid/1 -> can_signal_process_wio_validity/6
  ovrdflg/1 -> can_signal_process_wio_validity/7
  ovrdval/1 -> can_signal_process_wio_validity/8
  srvcflg/1 -> can_signal_process_wio_validity/9
  srvcval/1 -> can_signal_process_wio_validity/10
  Active_fault_flg/1 -> can_signal_process_wio_validity/3

== system inp/INP/HMI_signals/DefMaxACCSetSpeed ==
  signal_in : Inport
  msg_valid : Inport  Port=2
  Active_fault_flg : Inport  Port=3
  can_signal_process_wio_validity : inp/can_signal_process_wio_validity
  conv_gain_offset : Subsystem
  defval : Constant  Value=KINP_DefMaxACCSetSpeed_kph_defval
  invalid : Constant  Value=KINP_DefMaxACCSetSpeed_kph_invalid
  min_max_limit : Subsystem
  ovrdflg : Constant  Value=KINP_DefMaxACCSetSpeed_kph_ovrdflg
  ovrdval : Constant  Value=KINP_DefMaxACCSetSpeed_kph_ovrdval
  setvalid : Constant  Value=KINP_DefMaxACCSetSpeed_kph_setvalid
  srvcflg : Constant  Value=KINP_DefMaxACCSetSpeed_kph_srvcflg
  srvcval : Constant  Value=KINP_DefMaxACCSetSpeed_kph_srvcval
  signal_out : Outport
  signal_valid_out : Outport  Port=2
  wires:
  can_signal_process_wio_validity/1 -> min_max_limit/1(in)
  can_signal_process_wio_validity/2 -> signal_valid_out/1
  signal_in/1 -> conv_gain_offset/1(in)
  msg_valid/1 -> can_signal_process_wio_validity/2
  defval/1 -> can_signal_process_wio_validity/4
  invalid/1 -> can_signal_process_wio_validity/5
  conv_gain_offset/1(out) -> can_signal_process_wio_validity/1
  min_max_limit/1(out) -> signal_out/1
  setvalid/1 -> can_signal_process_wio_validity/6
  ovrdflg/1 -> can_signal_process_wio_validity/7
  ovrdval/1 -> can_signal_process_wio_validity/8
  srvcflg/1 -> can_signal_process_wio_validity/9
  srvcval/1 -> can_signal_process_wio_validity/10
  Active_fault_flg/1 -> can_signal_process_wio_validity/3

== system inp/INP/HMI_signals/FCWEnableH ==
  signal_in : Inport
  msg_valid : Inport  Port=2
  Active_fault_flg : Inport  Port=3
  can_signal_process_wio_validity : inp/can_signal_process_wio_validity
  defval : Constant  Value=KINP_FCWEnableH_flg_defval
  invalid : Constant  Value=KINP_FCWEnableH_flg_invalid
  ovrdflg : Constant  Value=KINP_FCWEnableH_flg_ovrdflg
  ovrdval : Constant  Value=KINP_FCWEnableH_flg_ovrdval
  setvalid : Constant  Value=KINP_FCWEnableH_flg_setvalid
  srvcflg : Constant  Value=KINP_FCWEnableH_flg_srvcflg
  srvcval : Constant  Value=KINP_FCWEnableH_flg_srvcval
  signal_out : Outport
  signal_valid_out : Outport  Port=2
  wires:
  Active_fault_flg/1 -> can_signal_process_wio_validity/3
  srvcval/1 -> can_signal_process_wio_validity/10
  srvcflg/1 -> can_signal_process_wio_validity/9
  ovrdval/1 -> can_signal_process_wio_validity/8
  ovrdflg/1 -> can_signal_process_wio_validity/7
  setvalid/1 -> can_signal_process_wio_validity/6
  invalid/1 -> can_signal_process_wio_validity/5
  defval/1 -> can_signal_process_wio_validity/4
  msg_valid/1 -> can_signal_process_wio_validity/2
  signal_in/1 -> can_signal_process_wio_validity/1
  can_signal_process_wio_validity/2 -> signal_valid_out/1
  can_signal_process_wio_validity/1 -> signal_out/1

== system inp/INP/HMI_signals/FCWWarningModeH ==
  signal_in : Inport
  msg_valid : Inport  Port=2
  Active_fault_flg : Inport  Port=3
  can_signal_process_wio_validity : inp/can_signal_process_wio_validity
  defval : Constant  Value=KINP_FCWWarningModeH_enum_defval
  invalid : Constant  Value=KINP_FCWWarningModeH_enum_invalid
  ovrdflg : Constant  Value=KINP_FCWWarningModeH_enum_ovrdflg
  ovrdval : Constant  Value=KINP_FCWWarningModeH_enum_ovrdval
  setvalid : Constant  Value=KINP_FCWWarningModeH_enum_setvalid
  srvcflg : Constant  Value=KINP_FCWWarningModeH_enum_srvcflg
  srvcval : Constant  Value=KINP_FCWWarningModeH_enum_srvcval
  signal_out : Outport
  signal_valid_out : Outport  Port=2
  wires:
  can_signal_process_wio_validity/1 -> signal_out/1
  can_signal_process_wio_validity/2 -> signal_valid_out/1
  signal_in/1 -> can_signal_process_wio_validity/1
  msg_valid/1 -> can_signal_process_wio_validity/2
  defval/1 -> can_signal_process_wio_validity/4
  invalid/1 -> can_signal_process_wio_validity/5
  setvalid/1 -> can_signal_process_wio_validity/6
  ovrdflg/1 -> can_signal_process_wio_validity/7
  ovrdval/1 -> can_signal_process_wio_validity/8
  srvcflg/1 -> can_signal_process_wio_validity/9
  srvcval/1 -> can_signal_process_wio_validity/10
  Active_fault_flg/1 -> can_signal_process_wio_validity/3

== system inp/INP/HMI_signals/IGSAOnH ==
  signal_in : Inport
  msg_valid : Inport  Port=2
  Active_fault_flg : Inport  Port=3
  can_signal_process_wio_validity : inp/can_signal_process_wio_validity
  defval : Constant  Value=KINP_IGSAOnH_flg_defval
  invalid : Constant  Value=KINP_IGSAOnH_flg_invalid
  ovrdflg : Constant  Value=KINP_IGSAOnH_flg_ovrdflg
  ovrdval : Constant  Value=KINP_IGSAOnH_flg_ovrdval
  setvalid : Constant  Value=KINP_IGSAOnH_flg_setvalid
  srvcflg : Constant  Value=KINP_IGSAOnH_flg_srvcflg
  srvcval : Constant  Value=KINP_IGSAOnH_flg_srvcval
  signal_out : Outport
  signal_valid_out : Outport  Port=2
  wires:
  can_signal_process_wio_validity/1 -> signal_out/1
  can_signal_process_wio_validity/2 -> signal_valid_out/1
  signal_in/1 -> can_signal_process_wio_validity/1
  msg_valid/1 -> can_signal_process_wio_validity/2
  defval/1 -> can_signal_process_wio_validity/4
  invalid/1 -> can_signal_process_wio_validity/5
  setvalid/1 -> can_signal_process_wio_validity/6
  ovrdflg/1 -> can_signal_process_wio_validity/7
  ovrdval/1 -> can_signal_process_wio_validity/8
  srvcflg/1 -> can_signal_process_wio_validity/9
  srvcval/1 -> can_signal_process_wio_validity/10
  Active_fault_flg/1 -> can_signal_process_wio_validity/3

== system inp/INP/HMI_signals/IGSAResSpdIncH ==
  signal_in : Inport
  msg_valid : Inport  Port=2
  Active_fault_flg : Inport  Port=3
  can_signal_process_wio_validity : inp/can_signal_process_wio_validity
  defval : Constant  Value=KINP_IGSAResSpdIncH_flg_defval
  invalid : Constant  Value=KINP_IGSAResSpdIncH_flg_invalid
  ovrdflg : Constant  Value=KINP_IGSAResSpdIncH_flg_ovrdflg
  ovrdval : Constant  Value=KINP_IGSAResSpdIncH_flg_ovrdval
  setvalid : Constant  Value=KINP_IGSAResSpdIncH_flg_setvalid
  srvcflg : Constant  Value=KINP_IGSAResSpdIncH_flg_srvcflg
  srvcval : Constant  Value=KINP_IGSAResSpdIncH_flg_srvcval
  signal_out : Outport
  signal_valid_out : Outport  Port=2
  wires:
  Active_fault_flg/1 -> can_signal_process_wio_validity/3
  srvcval/1 -> can_signal_process_wio_validity/10
  srvcflg/1 -> can_signal_process_wio_validity/9
  ovrdval/1 -> can_signal_process_wio_validity/8
  ovrdflg/1 -> can_signal_process_wio_validity/7
  setvalid/1 -> can_signal_process_wio_validity/6
  invalid/1 -> can_signal_process_wio_validity/5
  defval/1 -> can_signal_process_wio_validity/4
  msg_valid/1 -> can_signal_process_wio_validity/2
  signal_in/1 -> can_signal_process_wio_validity/1
  can_signal_process_wio_validity/2 -> signal_valid_out/1
  can_signal_process_wio_validity/1 -> signal_out/1

== system inp/INP/HMI_signals/IGSASetSpdDecH ==
  signal_in : Inport
  msg_valid : Inport  Port=2
  Active_fault_flg : Inport  Port=3
  can_signal_process_wio_validity : inp/can_signal_process_wio_validity
  defval : Constant  Value=KINP_IGSASetSpdDecH_flg_defval
  invalid : Constant  Value=KINP_IGSASetSpdDecH_flg_invalid
  ovrdflg : Constant  Value=KINP_IGSASetSpdDecH_flg_ovrdflg
  ovrdval : Constant  Value=KINP_IGSASetSpdDecH_flg_ovrdval
  setvalid : Constant  Value=KINP_IGSASetSpdDecH_flg_setvalid
  srvcflg : Constant  Value=KINP_IGSASetSpdDecH_flg_srvcflg
  srvcval : Constant  Value=KINP_IGSASetSpdDecH_flg_srvcval
  signal_out : Outport
  signal_valid_out : Outport  Port=2
  wires:
  can_signal_process_wio_validity/1 -> signal_out/1
  can_signal_process_wio_validity/2 -> signal_valid_out/1
  signal_in/1 -> can_signal_process_wio_validity/1
  msg_valid/1 -> can_signal_process_wio_validity/2
  defval/1 -> can_signal_process_wio_validity/4
  invalid/1 -> can_signal_process_wio_validity/5
  setvalid/1 -> can_signal_process_wio_validity/6
  ovrdflg/1 -> can_signal_process_wio_validity/7
  ovrdval/1 -> can_signal_process_wio_validity/8
  srvcflg/1 -> can_signal_process_wio_validity/9
  srvcval/1 -> can_signal_process_wio_validity/10
  Active_fault_flg/1 -> can_signal_process_wio_validity/3

== system inp/INP/HMI_signals/IGSASwitchModeH ==
  signal_in : Inport
  msg_valid : Inport  Port=2
  Active_fault_flg : Inport  Port=3
  can_signal_process_wio_validity : inp/can_signal_process_wio_validity
  defval : Constant  Value=KINP_IGSASwitchModeH_flg_defval
  invalid : Constant  Value=KINP_IGSASwitchModeH_flg_invalid
  ovrdflg : Constant  Value=KINP_IGSASwitchModeH_flg_ovrdflg
  ovrdval : Constant  Value=KINP_IGSASwitchModeH_flg_ovrdval
  setvalid : Constant  Value=KINP_IGSASwitchModeH_flg_setvalid
  srvcflg : Constant  Value=KINP_IGSASwitchModeH_flg_srvcflg
  srvcval : Constant  Value=KINP_IGSASwitchModeH_flg_srvcval
  signal_out : Outport
  signal_valid_out : Outport  Port=2
  wires:
  Active_fault_flg/1 -> can_signal_process_wio_validity/3
  srvcval/1 -> can_signal_process_wio_validity/10
  srvcflg/1 -> can_signal_process_wio_validity/9
  ovrdval/1 -> can_signal_process_wio_validity/8
  ovrdflg/1 -> can_signal_process_wio_validity/7
  setvalid/1 -> can_signal_process_wio_validity/6
  invalid/1 -> can_signal_process_wio_validity/5
  defval/1 -> can_signal_process_wio_validity/4
  msg_valid/1 -> can_signal_process_wio_validity/2
  signal_in/1 -> can_signal_process_wio_validity/1
  can_signal_process_wio_validity/2 -> signal_valid_out/1
  can_signal_process_wio_validity/1 -> signal_out/1

== system inp/INP/HMI_signals/LCSEnableH ==
  signal_in : Inport
  msg_valid : Inport  Port=2
  Active_fault_flg : Inport  Port=3
  can_signal_process_wio_validity : inp/can_signal_process_wio_validity
  defval : Constant  Value=KINP_LCSEnableH_flg_defval
  invalid : Constant  Value=KINP_LCSEnableH_flg_invalid
  ovrdflg : Constant  Value=KINP_LCSEnableH_flg_ovrdflg
  ovrdval : Constant  Value=KINP_LCSEnableH_flg_ovrdval
  setvalid : Constant  Value=KINP_LCSEnableH_flg_setvalid
  srvcflg : Constant  Value=KINP_LCSEnableH_flg_srvcflg
  srvcval : Constant  Value=KINP_LCSEnableH_flg_srvcval
  signal_out : Outport
  signal_valid_out : Outport  Port=2
  wires:
  Active_fault_flg/1 -> can_signal_process_wio_validity/3
  srvcval/1 -> can_signal_process_wio_validity/10
  srvcflg/1 -> can_signal_process_wio_validity/9
  ovrdval/1 -> can_signal_process_wio_validity/8
  ovrdflg/1 -> can_signal_process_wio_validity/7
  setvalid/1 -> can_signal_process_wio_validity/6
  invalid/1 -> can_signal_process_wio_validity/5
  defval/1 -> can_signal_process_wio_validity/4
  msg_valid/1 -> can_signal_process_wio_validity/2
  signal_in/1 -> can_signal_process_wio_validity/1
  can_signal_process_wio_validity/2 -> signal_valid_out/1
  can_signal_process_wio_validity/1 -> signal_out/1

== system inp/INP/HMI_signals/LFCPilotCancelH ==
  signal_in : Inport
  msg_valid : Inport  Port=2
  Active_fault_flg : Inport  Port=3
  can_signal_process_wio_validity : inp/can_signal_process_wio_validity
  defval : Constant  Value=KINP_LFCPilotCancelH_flg_defval
  invalid : Constant  Value=KINP_LFCPilotCancelH_flg_invalid
  ovrdflg : Constant  Value=KINP_LFCPilotCancelH_flg_ovrdflg
  ovrdval : Constant  Value=KINP_LFCPilotCancelH_flg_ovrdval
  setvalid : Constant  Value=KINP_LFCPilotCancelH_flg_setvalid
  srvcflg : Constant  Value=KINP_LFCPilotCancelH_flg_srvcflg
  srvcval : Constant  Value=KINP_LFCPilotCancelH_flg_srvcval
  signal_out : Outport
  signal_valid_out : Outport  Port=2
  wires:
  Active_fault_flg/1 -> can_signal_process_wio_validity/3
  srvcval/1 -> can_signal_process_wio_validity/10
  srvcflg/1 -> can_signal_process_wio_validity/9
  ovrdval/1 -> can_signal_process_wio_validity/8
  ovrdflg/1 -> can_signal_process_wio_validity/7
  setvalid/1 -> can_signal_process_wio_validity/6
  invalid/1 -> can_signal_process_wio_validity/5
  defval/1 -> can_signal_process_wio_validity/4
  msg_valid/1 -> can_signal_process_wio_validity/2
  signal_in/1 -> can_signal_process_wio_validity/1
  can_signal_process_wio_validity/2 -> signal_valid_out/1
  can_signal_process_wio_validity/1 -> signal_out/1
